simulink model "ROV_Simulation"  (R2024b)
settings: Solver=ode15s  StartTime=0.0  StopTime=120  MaxStep=0.01  MinStep=0.0001  RelTol=1e-2
PreLoadFcn:
  | load('Simulation_busses.mat')
  | 
  | %Load Initicial Conditions
  | load("InitCond.mat");
  | nu_Init = [nu_IC.u nu_IC.v nu_IC.w nu_IC.p nu_IC.q nu_IC.r];
  | eta_Init = [eta_IC.N eta_IC.E eta_IC.D eta_IC.phi eta_IC.theta eta_IC.psi];
  | 
  | %Load simulator preferences
  | load("simparam.mat");
  | %Load Thruster settings
  | load("TAM.mat");
  | load("thrusterLimit.mat");
  | %Load External forces
  | load("External_forces.mat");
  | %Load sensors
  | load("Dependencies\Workspaces\Sensors.mat");
PostLoadFcn:
  | %Define Mask for Equation of Motion
  | mask = Simulink.Mask.get('ROV_Simulation/Equation of Motion');
  | 
  | %Set options for Robots
  | robots = mask.getParameter('Robots');
  | robots.TypeOptions = who("-file","Robots.mat");
  | 
  | %Set Options for Environments
  | environments = mask.getParameter('Environments');
  | environments.TypeOptions = who("-file","Environments.mat");
  | 
  | %Set path to VR World in VR Tracer
  | %set_param('ROV_Simulation/eta2VR/VR Tracer','VrmlFile',which('Good_world_BlueRov.x3d'));
  | %set_param('ROV_Simulation/Reference Generator/VR Tracer','VrmlFile',which('Good_world_BlueRov.x3d'));
  | %set_param('ROV_Simulation/VR Sink','WorldFileName',which('Good_world_BlueRov.x3d'))
InitFcn:
  | % load local location of 3d world
  | vrFile = fullfile(pwd, 'Dependencies', 'Animation', 'Good_world_BlueRov.x3d'); % Construct the path
  | set_param('ROV_Simulation/VR Sink', 'WorldFileName', vrFile); % Update the block's parameter
  | 
  | load('Simulation_busses.mat');
  | %Load simulator preferences
  | load("simparam.mat");
  | 
  | %Load Thruster settings
  | load("TAM.mat");
  | load("thrusterLimit.mat");
  | 
  | %Load External forces
  | load("External_forces.mat");
  | 
  | %Load Reference Case study
  | load("Reference_trajectory_case_study.mat");
  | 
  | % COMMENTED OUT BY ETU 13/8-24
  | %if(not(isMATLABReleaseOlderThan("R2021b")))
  | %set_param('ROV_Simulation/External Forces/Tether','commented',double(not(Sim_param.extTetUse)))
  | %set_param('ROV_Simulation/External Forces/Manual disturbance','commented',double(not(Sim_param.extManUse)))
  | %end
  | 
  | %Load sensors
  | load("Dependencies\Workspaces\Sensors.mat");
  | noise = NoiseSelection();
  | 
  | %Load Initicial Conditions
  | load("InitCond.mat");
  | nu_Init = [nu_IC.u nu_IC.v nu_IC.w nu_IC.p nu_IC.q nu_IC.r];
  | eta_Init = [eta_IC.N eta_IC.E eta_IC.D eta_IC.phi eta_IC.theta eta_IC.psi];
  | 
  | % Load Physical parameters:
  | if(get_param('ROV_Simulation/Equation of Motion','use_app_parameters') == "on")
  |     %env = get_param('ROV_Simulation/Equation of Motion','Environments');
  |     %env = str2sym(env);
  |     %env = sprintf("%s",env);
  |     Env = LoadEnvironment(Sim_param.Env);
  | end
  | 
  | % Load robot chosen in "Equation of Motion" block:
  | if(get_param('ROV_Simulation/Equation of Motion','use_app_parameters') == "on")
  |     %rob = get_param('ROV_Simulation/Equation of Motion','Robots');
  |     %rob = str2sym(rob);
  |     %rob = sprintf("%s",rob);
  |     Robot = LoadRobotParameters(Sim_param.Robot);
  | else
  |     ROV_Constants;
  |     Robot = ROV_Struct2Bus(ROV);
  | end
  | 
  | % Pos Rov
  | xi = eta_Init(1);
  | yi = eta_Init(2);
  | zi = eta_Init(3);
  | 
  | knots = 11;
  | len_tet = 35;
  | ln_tet = len_tet/(knots-1);
  | z_tet_i = 0;
  | z1 = zi/2-ln_tet;
  | 
  | len_1 = len_tet/2-ln_tet;
  | x_tet=zeros(knots,1);
  | z_tet=zeros(knots,1);
  | if zi == len_tet
  |     z_tet = linspace(0,zi,knots)';
  | else
  | x1 = sqrt(len_1^2-z1^2);
  | a1 = (z1-z_tet_i)/(x1-0);
  | x_inc = x1/((knots-1)/2-1);
  | 
  | for i=2:1:(knots-1)/2
  | x_tet(i,1) = x_inc*(i-1);
  | end
  | z_tet(1:(knots-1)/2,1) = a1*x_tet(1:(knots-1)/2,1);
  | z2 = zi/2+ln_tet ;
  | x2 = sqrt(len_1^2-z1^2);
  | a2 = (zi-z2)/(0-x2);
  | x_tet((knots+1)/2:(knots-1)/2+2,1) = x2;
  | z_tet((knots+1)/2) = z2-ln_tet;
  | for i=(knots-1)/2+2:1:knots-1
  | x_tet(i+1,1) = x2-x_inc*(i-((knots+1)/2));
  | end
  | x_tet((knots-1)/2+1:(knots-1)/2+2,1) = x2;
  | z_tet((knots+1)/2+1:end,1) = a2*x_tet((knots+1)/2+1:end,1)+zi;
  | end
  | x_tet = x_tet+xi;
  | P10=[xi yi zi]';
  | P9=[x_tet(10) yi z_tet(10) ]';
  | P8=[x_tet(9) yi z_tet(9) ]';
  | P7=[x_tet(8)  yi z_tet(8) ]';
  | P6=[x_tet(7) yi z_tet(7)]';
  | P5=[(x_tet(6)) yi z_tet(6)]';
  | P4=[x_tet(5) yi z_tet(5)]';
  | P3=[x_tet(4) yi z_tet(4)]';
  | P2=[x_tet(3) yi z_tet(3)]';
  | P1=[x_tet(2) yi z_tet(2)]';
  | P0=[xi yi 0]';
  | 
  | 
  | x0 = 0;
  | y0 = -5;
  | z0 = 5;
  | Vr = 0.5;
  | R = 10;
  | total_time = 120;
  | dt = 0.0001;
  | 
  | [path, t_path] = pathgen(Vr, R, x0, y0, z0, total_time, dt);
  | 
  | % Enregistrer séparément
  | save('C:\Users\smaha\OneDrive\Bureau\ROVSimulator2024a\Parameters\path.mat', 'path');
  | save('C:\Users\smaha\OneDrive\Bureau\ROVSimulator2024a\Parameters\t_path.mat', 't_path');
  | 
  | % Charger dans l’espace de travail de base
  | %tmp1 = load('C:\Users\smaha\OneDrive\Bureau\ROVSimulator2024a\Parameters\path.mat');
  | %tmp2 = load('C:\Users\smaha\OneDrive\Bureau\ROVSimulator2024a\Parameters\t_path.mat');
  | 
  | %assignin('base', 'path', tmp1.path);
  | %assignin('base', 't_path', tmp2.t_path);
undefined symbols (not defined in the file or in its folder): eta_IC, EXT, nu_IC, Sim_param, T, T_inv, thrustLowerLimit, thrustUpperLimit
libraries: vrlib x2
(part 1 of 2)
settings Model: Creator=GT82BC  LastModifiedBy=smaha
settings SolverCC: InitialStep=0.0001  MaxOrder=2  MaxConsecutiveZCs=10^6  MaxConsecutiveMinStep=100  AlgebraicLoopSolver=TrustRegion
settings DebuggingCC: InheritedTsInSrcMsg=none  UnconnectedInputMsg=warning  UnconnectedOutputMsg=warning  UnconnectedLineMsg=warning  BusObjectLabelMismatch=none
settings ModelReferenceCC: EnableRefExpFcnMdlSchedulingChecks=on
settings RTWCC: GPUComputeCapability=3.5  DLArmComputeVersion=19.05
settings ConfigComp: CovScope=ReferencedModels

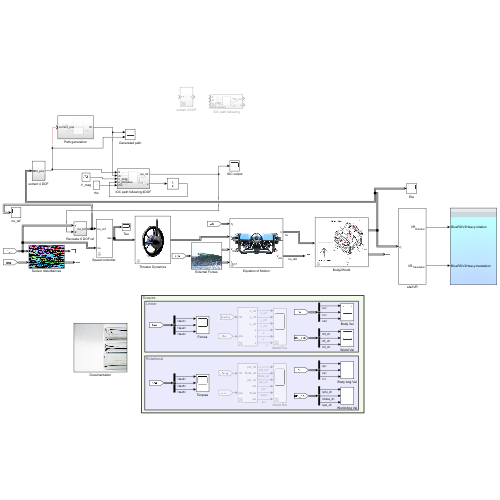
[thumbnail: Simulink's stored preview of the top-level diagram]
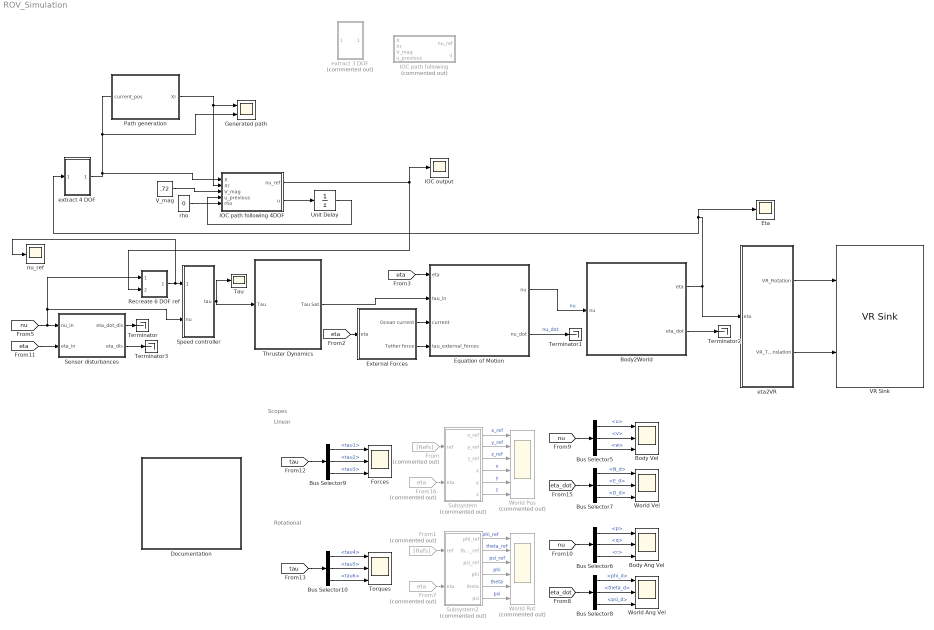
== system ROV_Simulation ==
  note: Rotational
  note: Linear
  note: Scopes
  Body Ang Vel : Scope  NumInputPorts=3
  Body Vel : Scope  NumInputPorts=3
  Body2World : Subsystem  mask(Body-Fixed to Earth-Fixed){InitialCondition=eta_Init}
      mask prompts: InitialCondition "Simulink:blkprm_prompts:IntegratorInitCond"
      mask Help: winopen('Documentation\ROVmodel.pdf')
      mask DialogControl InitialCondition Prompt: Initial condition:
      mask Display: image('BlueROV1.png')
  Bus Selector10 : BusSelector  OutputSignals=tau4,tau5,tau6
  Bus Selector5 : BusSelector  OutputSignals=u,v,w
  Bus Selector6 : BusSelector  OutputSignals=p,q,r
  Bus Selector7 : BusSelector  OutputSignals=N_d,E_d,D_d
  Bus Selector8 : BusSelector  OutputSignals=phi_d,theta_d,psi_d
  Bus Selector9 : BusSelector  OutputSignals=tau1,tau2,tau3
  Documentation : Subsystem
      settings: OpenFcn="open(\"Documentation\\ROVmodel.pdf\");"
      mask Display: image('documentation.jpg'); % Use "Edit Image" on toolstrip to change.
  Equation of Motion : Subsystem  mask(Equation of Motion){InitialCondition=nu_Init, use_app_parameters=on, Robots=BlueROV2_Heavy, Environments=Offshore}
      mask prompts: InitialCondition "Simulink:blkprm_prompts:IntegratorInitCond"; use_app_parameters "Use parameters from App"; Robots "Robot"; Environments "Environment"
      mask init:
      | mask = Simulink.Mask.get('ROV_Simulation/Equation of Motion');
      | btn = mask.getDialogControl('mscript_btn');
      | app_panel = mask.getDialogControl('app_parameter_panel');
      | mscript_panel = mask.getDialogControl('mscript_parameter_panel');
      | if(get_param('ROV_Simulation/Equation of Motion','use_app_parameters') == "off")
      |     app_panel.Visible = 'off';
      |     mscript_panel.Visible = 'on';
      | else
      |     app_panel.Visible = 'on';
      |     mscript_panel.Visible = 'off';
      | end
      mask TypeOptions InitialCondition: Integrator/InitialCondition
      mask Callback use_app_parameters:
      | mask = Simulink.Mask.get('ROV_Simulation/Equation of Motion');
      | btn = mask.getDialogControl('mscript_btn');
      | app_panel = mask.getDialogControl('app_parameter_panel');
      | mscript_panel = mask.getDialogControl('mscript_parameter_panel');
      | if(get_param('ROV_Simulation/Equation of Motion','use_app_parameters') == "off")
      |     app_panel.Visible = 'off';
      |     mscript_panel.Visible = 'on';
      | else
      |     app_panel.Visible = 'on';
      |     mscript_panel.Visible = 'off';
      | end
      mask TypeOptions Robots: BlueROV2_Heavy
      mask TypeOptions Environments: Lab | Offshore
      mask DialogControl InitialCondition Prompt: Initial condition:
      mask DialogControl refresh_options Prompt: Refresh Options
      mask DialogControl refresh_options Callback:
      | %Define Mask for Equation of Motion
      | mask = Simulink.Mask.get('ROV_Simulation/Equation of Motion');
      | 
      | %Set options for Robots
      | robots = mask.getParameter('Robots');
      | robots.TypeOptions = who("-file","Robots.mat");
      | 
      | %Set Options for Environments
      | environments = mask.getParameter('Environments');
      | environments.TypeOptions = who("-file","Environments.mat");
      mask DialogControl Robot_script_btn Prompt: ROV Parameters
      mask DialogControl Robot_script_btn Callback: open('ROV_Constants.m');
      mask DialogControl Environment_script_btn Prompt: Environmental Parameters
      mask DialogControl Environment_script_btn Callback: open('Environmental_Constants.m');
      mask DialogControl ChangeGroup Prompt: Change Parameters
      mask DialogControl Change_Parameters Prompt: Open App
      mask DialogControl Change_Parameters Callback: ROV_App;
      mask Display:
      | image('$imagefile'); % Use "Edit Image" on toolstrip to change.
      | 
      | port_label('input',1,'\eta','texmode', 'on')
      | port_label('input',2,'\tau_{in}','texmode', 'on')
      | port_label('input',3,'\nu_{Water}','texmode', 'on')
      | port_label('input',4,'\tau_{EXT}','texmode', 'on')
      | 
      | 
      | port_label('output',1,'\nu','texmode', 'on')
      | port_label('output',2,'\nu_{dot}','texmode','on')
  Eta : Scope
  External Forces : Subsystem
      mask Display: image('Waves.jpg')
  Forces : Scope  NumInputPorts=3
  From : From  Commented=on  GotoTag=Refs
  From1 : From  Commented=on  GotoTag=Refs
  From16 : From  Commented=on  GotoTag=eta  TagVisibility=global
  From7 : From  Commented=on  GotoTag=eta  TagVisibility=global
  Generated path : Scope  NumInputPorts=2
  IOC output : Scope
  IOC path following : Subsystem  Commented=on
  IOC path following 4DOF : Subsystem
  Path generation : Subsystem
  Recreate 6 DOF ref : Subsystem
  Sensor disturbances : Subsystem
      mask Display: image('Noise.jpg')
  "Speed controller " : Subsystem  mask{Kp=10, Ki=10, Kd=0}
  Subsystem : Subsystem  Commented=on
  Subsystem2 : Subsystem  Commented=on
  Tau : Scope
  Terminator{,1..3} (4 alike) : Terminator
  Thruster Dynamics : Subsystem  mask(Thruster Dynamics){}
      mask help: Thruster Dynamics & Saturation
      mask Display: image('Thruster11.jpg')
  Torques : Scope  NumInputPorts=3
  Unit Delay : UnitDelay  SampleTime=-1  HasFrameUpgradeWarning=on
  VR Sink : vrlib/VR Sink  InstantiateOnLoad=on  SampleTime=0.1  ViewEnable=on  RemoteChange=off  RemoteView=off  FieldsWritten=BlueROV2Heavy.rotation.4.1.1.double#BlueROV2Heavy.translation.3.1.1.double  WorldFileName=C:\Users\smaha\OneDrive\Bureau\ROVSimulator2024a\Dependencies\Animation\Good_world_BlueRov.x3d  AutoView=on  VideoDimensions=[]  AllowVariableSize=off  FigureProperties="{'Position', 'Name', 'Viewpoint', 'CameraBound', 'CameraDirection', 'CameraPosition', 'CameraUpVector', 'ZoomFactor', 'Antialiasing', 'Headlight', 'Lighting', 'Sound', 'StatusBar', 'Stereo3D', 'Stereo3DCameraOffset', 'Stereo3DHIT', 'ToolBar', 'Textures', 'Transparency', 'Triad', 'Wireframe', 'NavZones', 'NavMode', 'NavSpeed', 'CaptureFileFormat', 'CaptureFileName', 'Record2DCompressMethod', 'Record2DCompressQuality', 'Record2D', 'Record2DFileName', 'Record2DFPS', 'NavPanel'; [1.0000000000000000e+00   7.4199999999999932e+01   1.5360000000000000e+03   6.9080000000000007e+02], '', 'FixedtoROV', 'on', [3.1410759951924716e-02   0.0000000000000000e+00  -9.9950656033827134e-01], [3.7692911942309659e-01   0.0000000000000000e+00   2.0059212759407377e+00], [0.0000000000000000e+00   1.0000000000000000e+00   0.0000000000000000e+00], [1.0000000000000000e+00], 'on', 'on', 'on', 'on', 'on', 'off', [1.0000000149011612e-01], [0.0000000000000000e+00], 'on', 'on', 'on', 'bottomright', 'off', 'off', 'walk', 'normal', 'tif', '%f_anim_%n.tif', 'auto', [7.5000000000000000e+01], 'off', '%f_anim_%n.avi', 'auto', 'halfbar'}"
  V_mag : Constant  Value=.72
  World Ang Vel : Scope  NumInputPorts=3
  World Pos : Scope  Commented=on  NumInputPorts=6
  World Rot : Scope  Commented=on  NumInputPorts=6
  World Vel : Scope  NumInputPorts=3
  eta2VR : Subsystem
      mask Display:
      | port_label('input',1,'\eta','texmode', 'on')
      | 
      | port_label('output',1,'VR_{Rotation}','texmode', 'on')
      | port_label('output',2,'VR_{Translation}','texmode','on')
  extract 3 DOF : Subsystem  Commented=on
  extract 4 DOF : Subsystem
  nu_ref : Scope
  rho : Constant  Value=0
  wires:
  Equation of Motion/2(nu_dot) -> Terminator1/1  "nu_dot"
  Bus Selector8/1 -> World Ang Vel/1  "<phi_d>"
  Bus Selector10/2 -> Torques/2  "<tau5>"
  Bus Selector8/2 -> World Ang Vel/2  "<theta_d>"
  [tau] -> Bus Selector9/1
  Bus Selector8/3 -> World Ang Vel/3  "<psi_d>"
  [nu] -> Bus Selector6/1
  Bus Selector5/3 -> Body Vel/3  "<w>"
  Bus Selector10/3 -> Torques/3  "<tau6>"
  [eta_dot] -> Bus Selector8/1
  Bus Selector7/1 -> World Vel/1  "<N_d>"
  [eta_dot] -> Bus Selector7/1
  Bus Selector6/3 -> Body Ang Vel/3  "<r>"
  Bus Selector6/1 -> Body Ang Vel/1  "<p>"
  Bus Selector5/1 -> Body Vel/1  "<u>"
  Bus Selector7/3 -> World Vel/3  "<D_d>"
  Bus Selector5/2 -> Body Vel/2  "<v>"
  Bus Selector9/2 -> Forces/2  "<tau2>"
  Bus Selector7/2 -> World Vel/2  "<E_d>"
  Bus Selector10/1 -> Torques/1  "<tau4>"
  [nu] -> Bus Selector5/1
  Bus Selector6/2 -> Body Ang Vel/2  "<q>"
  Bus Selector9/1 -> Forces/1  "<tau1>"
  Bus Selector9/3 -> Forces/3  "<tau3>"
  From16/1 -> Subsystem/2(eta)
  [tau] -> Bus Selector10/1
  From/1 -> Subsystem/1(ref)
  Subsystem/1(x_ref) -> World Pos/1  "x_ref"
  Subsystem/2(y_ref) -> World Pos/2  "y_ref"
  Subsystem/3(z_ref) -> World Pos/3  "z_ref"
  Subsystem/4(x) -> World Pos/4  "x"
  Subsystem/5(y) -> World Pos/5  "y"
  Subsystem/6(z) -> World Pos/6  "z"
  From7/1 -> Subsystem2/2(eta)
  From1/1 -> Subsystem2/1(ref)
  Subsystem2/1(phi_ref) -> World Rot/1  "phi_ref"
  Subsystem2/2(theta_ref) -> World Rot/2  "theta_ref"
  Subsystem2/3(psi_ref) -> World Rot/3  "psi_ref"
  Subsystem2/4(phi) -> World Rot/4  "phi"
  Subsystem2/5(theta) -> World Rot/5  "theta"
  Subsystem2/6(psi) -> World Rot/6  "psi"
  [eta] -> Equation of Motion/1(eta)
  [eta] -> Sensor disturbances/2(eta_in)
  eta2VR/1(VR_Rotation) -> VR Sink/1
  eta2VR/2(VR_Translation) -> VR Sink/2
  Thruster Dynamics/1(Tau Sat) -> Equation of Motion/2(tau_in)
  [eta] -> External Forces/1(eta)
  External Forces/1(Ocean current) -> Equation of Motion/3(current)
  External Forces/2(Tether force) -> Equation of Motion/4(tau_external_forces)
  Equation of Motion/1(nu) -> Body2World/1(nu)  "nu"
  Body2World/2(eta_dot) -> Terminator2/1
  Body2World/1(eta) -> eta2VR/1(eta), Eta/1, extract 4 DOF/1(In1)
  V_mag/1 -> IOC path following 4DOF/3(V_mag)
  Path generation/1(Xr) -> IOC path following 4DOF/2(Xr), Generated path/1
  extract 4 DOF/1(current_pos) -> Generated path/2, IOC path following 4DOF/1(X)
  IOC path following 4DOF/1(nu_ref) -> IOC output/1, Recreate 6 DOF ref/2(In2)
  Recreate 6 DOF ref/1(nu_ref) -> nu_ref/1, "Speed controller "/1(nu_ref)
  IOC path following 4DOF/2(u) -> Unit Delay/1
  Unit Delay/1 -> IOC path following 4DOF/4(u_previous)
  Sensor disturbances/1(eta_dot_dis) -> Terminator/1
  "Speed controller "/1(tau) -> Thruster Dynamics/1(Tau), Tau/1
  [nu] -> Recreate 6 DOF ref/1(In1), "Speed controller "/2(nu), Sensor disturbances/1(nu_in)
  Sensor disturbances/2(eta_dis) -> Terminator3/1
  rho/1 -> IOC path following 4DOF/5(rho)

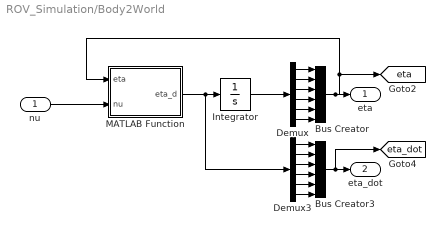
== system ROV_Simulation/Body2World ==
  nu : Inport  OutDataTypeStr=Bus: nu
  Bus Creator : BusCreator  Inputs=6  OutDataTypeStr=Bus: eta
      settings: InheritFromInputs=off
  Bus Creator3 : BusCreator  Inputs=6  OutDataTypeStr=Bus: eta_d
      settings: InheritFromInputs=off
  Demux : Demux  Outputs=6
  Demux3 : Demux  Outputs=6
  Integrator : Integrator  InitialCondition=InitialCondition
  MATLAB Function : MATLAB Function  TreatAsAtomicUnit=on
  eta : Outport  OutDataTypeStr=Bus: eta
  eta_dot : Outport  Port=2  OutDataTypeStr=Bus: eta_d
  tag scope: eta=global, eta_dot=global
  wires:
  Demux3/3 -> Bus Creator3/3
  MATLAB Function/1(eta_d) -> Demux3/1, Integrator/1
  Demux3/4 -> Bus Creator3/4
  Demux/4 -> Bus Creator/4
  Demux/5 -> Bus Creator/5
  Demux/1 -> Bus Creator/1
  Integrator/1 -> Demux/1
  Demux/3 -> Bus Creator/3
  Demux3/1 -> Bus Creator3/1
  Demux3/6 -> Bus Creator3/6
  Demux/6 -> Bus Creator/6
  Demux3/2 -> Bus Creator3/2
  Demux/2 -> Bus Creator/2
  Demux3/5 -> Bus Creator3/5
  Bus Creator/1 -> [eta], MATLAB Function/1(eta), eta/1
  Bus Creator3/1 -> [eta_dot], eta_dot/1
  nu/1 -> MATLAB Function/2(nu)

== system ROV_Simulation/Documentation ==

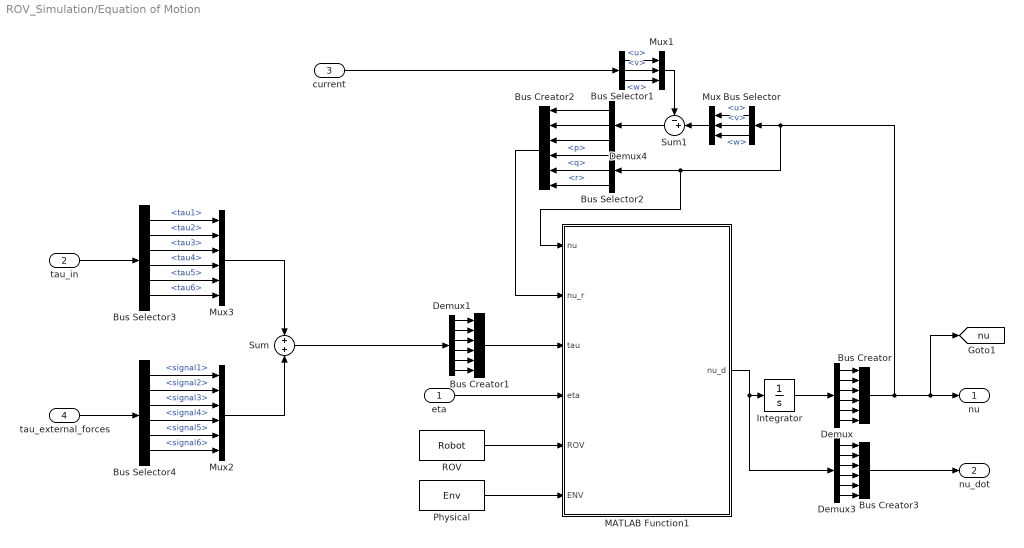
== system ROV_Simulation/Equation of Motion ==
  eta : Inport  OutDataTypeStr=Bus: eta
  tau_in : Inport  Port=2  OutDataTypeStr=Bus: tau
  current : Inport  Port=3  OutDataTypeStr=Bus: V_water
  tau_external_forces : Inport  Port=4  OutDataTypeStr=Bus: tau
  Bus Creator : BusCreator  Inputs=6  OutDataTypeStr=Bus: nu
      settings: InheritFromInputs=off
  Bus Creator1 : BusCreator  Inputs=6  OutDataTypeStr=Bus: tau
      settings: InheritFromInputs=off
  Bus Creator2 : BusCreator  Inputs=6  OutDataTypeStr=Bus: nu
      settings: InheritFromInputs=off
  Bus Creator3 : BusCreator  Inputs=6  OutDataTypeStr=Bus: nu_d
      settings: InheritFromInputs=off
  Bus Selector : BusSelector  OutputSignals=u,v,w
  Bus Selector1 : BusSelector  OutputSignals=u,v,w
  Bus Selector2 : BusSelector  OutputSignals=p,q,r
  Bus Selector3 : BusSelector  OutputSignals=tau1,tau2,tau3,tau4,tau5,tau6
  Bus Selector4 : BusSelector  OutputSignals=signal1,signal2,signal3,signal4,signal5,signal6
  Demux : Demux  Outputs=6
  Demux1 : Demux  Outputs=6
  Demux3 : Demux  Outputs=6
  Demux4 : Demux  Outputs=3
  Integrator : Integrator  InitialCondition=InitialCondition
  MATLAB Function1 : MATLAB Function  TreatAsAtomicUnit=on
  Mux : Mux  Inputs=3
  Mux1 : Mux  Inputs=3
  Mux2 : Mux  Inputs=6
  Mux3 : Mux  Inputs=6
  Physical : Constant  Value=Env  OutDataTypeStr=Bus: ENV_bus
  ROV : Constant  Value=Robot  OutDataTypeStr=Bus: ROV_bus
  Sum : Sum
  Sum1 : Sum  Inputs=-+|
  nu : Outport  OutDataTypeStr=Bus: nu
  nu_dot : Outport  Port=2  OutDataTypeStr=Bus: nu_d
  tag scope: nu=global
  wires:
  Demux3/3 -> Bus Creator3/3
  Demux/4 -> Bus Creator/4
  Demux3/4 -> Bus Creator3/4
  MATLAB Function1/1(nu_d) -> Demux3/1, Integrator/1
  Demux3/1 -> Bus Creator3/1
  Demux/5 -> Bus Creator/5
  Demux3/6 -> Bus Creator3/6
  Bus Creator3/1 -> nu_dot/1
  Physical/1 -> MATLAB Function1/6(ENV)
  Demux3/2 -> Bus Creator3/2
  Demux3/5 -> Bus Creator3/5
  Demux/2 -> Bus Creator/2
  Integrator/1 -> Demux/1
  Demux/1 -> Bus Creator/1
  Demux/3 -> Bus Creator/3
  Demux/6 -> Bus Creator/6
  eta/1 -> MATLAB Function1/4(eta)
  ROV/1 -> MATLAB Function1/5(ROV)
  Bus Creator1/1 -> MATLAB Function1/3(tau)
  Demux1/5 -> Bus Creator1/5
  Demux1/6 -> Bus Creator1/6
  Demux1/1 -> Bus Creator1/1
  Demux1/2 -> Bus Creator1/2
  Demux1/3 -> Bus Creator1/3
  Demux1/4 -> Bus Creator1/4
  Bus Creator/1 -> MATLAB Function1/1(nu), Bus Selector2/1, Bus Selector/1, [nu], nu/1
  Mux/1 -> Sum1/2
  Sum1/1 -> Demux4/1
  Demux4/1 -> Bus Creator2/1
  Demux4/2 -> Bus Creator2/2
  Demux4/3 -> Bus Creator2/3
  Bus Selector1/1 -> Mux1/1  "<u>"
  Bus Selector1/2 -> Mux1/2  "<v>"
  Bus Selector1/3 -> Mux1/3  "<w>"
  Mux1/1 -> Sum1/1
  Bus Selector/1 -> Mux/1  "<u>"
  Bus Selector/2 -> Mux/2  "<v>"
  Bus Selector/3 -> Mux/3  "<w>"
  current/1 -> Bus Selector1/1
  Bus Selector2/3 -> Bus Creator2/6  "<r>"
  Bus Selector2/2 -> Bus Creator2/5  "<q>"
  Bus Selector2/1 -> Bus Creator2/4  "<p>"
  Bus Creator2/1 -> MATLAB Function1/2(nu_r)
  tau_in/1 -> Bus Selector3/1
  tau_external_forces/1 -> Bus Selector4/1
  Bus Selector4/1 -> Mux2/1  "<signal1>"
  Bus Selector4/2 -> Mux2/2  "<signal2>"
  Bus Selector4/3 -> Mux2/3  "<signal3>"
  Bus Selector4/4 -> Mux2/4  "<signal4>"
  Bus Selector4/5 -> Mux2/5  "<signal5>"
  Bus Selector4/6 -> Mux2/6  "<signal6>"
  Bus Selector3/1 -> Mux3/1  "<tau1>"
  Bus Selector3/2 -> Mux3/2  "<tau2>"
  Bus Selector3/3 -> Mux3/3  "<tau3>"
  Bus Selector3/4 -> Mux3/4  "<tau4>"
  Bus Selector3/5 -> Mux3/5  "<tau5>"
  Bus Selector3/6 -> Mux3/6  "<tau6>"
  Mux3/1 -> Sum/1
  Mux2/1 -> Sum/2
  Sum/1 -> Demux1/1

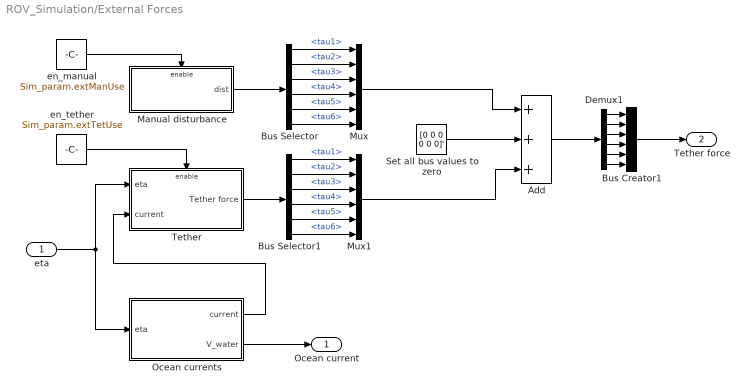
== system ROV_Simulation/External Forces ==
  eta : Inport
  Add : Sum  Inputs=+++
  Bus Creator1 : BusCreator  Inputs=6  OutDataTypeStr=Bus: tau
  Bus Selector : BusSelector  OutputSignals=tau1,tau2,tau3,tau4,tau5,tau6
  Bus Selector1 : BusSelector  OutputSignals=tau1,tau2,tau3,tau4,tau5,tau6
  Demux1 : Demux  Outputs=6
  Manual disturbance : Subsystem  TreatAsAtomicUnit=on
  Mux : Mux  Inputs=6
  Mux1 : Mux  Inputs=6
  Ocean currents : Subsystem  TreatAsAtomicUnit=on
  Set all bus values to zero : Constant  Value=[0 0 0 0 0 0]'
  Tether : Subsystem  TreatAsAtomicUnit=on
  en_manual : Constant  Value=Sim_param.extManUse
  en_tether : Constant  Value=Sim_param.extTetUse
  Ocean current : Outport
  Tether force : Outport  Port=2
  wires:
  eta/1 -> Ocean currents/1(eta), Tether/1(eta)
  Manual disturbance/1(dist) -> Bus Selector/1
  Demux1/4 -> Bus Creator1/4
  Demux1/2 -> Bus Creator1/2
  Demux1/5 -> Bus Creator1/5
  Demux1/1 -> Bus Creator1/1
  Bus Creator1/1 -> Tether force/1
  Demux1/6 -> Bus Creator1/6
  Demux1/3 -> Bus Creator1/3
  Ocean currents/1(current) -> Tether/2(current)
  Ocean currents/2(V_water) -> Ocean current/1
  en_tether/1 -> Tether/Enable
  en_manual/1 -> Manual disturbance/Enable
  Tether/1(Tether force) -> Bus Selector1/1
  Bus Selector1/1 -> Mux1/1  "<tau1>"
  Bus Selector1/2 -> Mux1/2  "<tau2>"
  Bus Selector1/3 -> Mux1/3  "<tau3>"
  Bus Selector1/4 -> Mux1/4  "<tau4>"
  Bus Selector1/5 -> Mux1/5  "<tau5>"
  Bus Selector1/6 -> Mux1/6  "<tau6>"
  Bus Selector/1 -> Mux/1  "<tau1>"
  Bus Selector/2 -> Mux/2  "<tau2>"
  Bus Selector/3 -> Mux/3  "<tau3>"
  Bus Selector/4 -> Mux/4  "<tau4>"
  Bus Selector/5 -> Mux/5  "<tau5>"
  Bus Selector/6 -> Mux/6  "<tau6>"
  Set all bus values to zero/1 -> Add/2
  Mux/1 -> Add/1
  Mux1/1 -> Add/3
  Add/1 -> Demux1/1

== system ROV_Simulation/IOC path following ==
  X : Inport
  Xr : Inport  Port=2
  V_mag : Inport  Port=3
  u_previous : Inport  Port=4
  IOC path following : MATLAB Function  TreatAsAtomicUnit=on
  Scope : Scope  NumInputPorts=2
  nu_ref : Outport
  u : Outport  Port=2
  wires:
  IOC path following/1(nu_ref) -> nu_ref/1
  X/1 -> IOC path following/1(X)
  Xr/1 -> IOC path following/2(Xr)
  V_mag/1 -> IOC path following/3(V_mag)
  u_previous/1 -> IOC path following/4(u_previous)
  IOC path following/2(u) -> u/1
  IOC path following/3(e2) -> Scope/1
  IOC path following/4(e3) -> Scope/2

== system ROV_Simulation/IOC path following 4DOF ==
  X : Inport
  Xr : Inport  Port=2
  V_mag : Inport  Port=3
  u_previous : Inport  Port=4
  rho : Inport  Port=5
  Errors : Scope  NumInputPorts=3
  IOC path following 4 DOF : MATLAB Function  TreatAsAtomicUnit=on
  nu_ref : Outport
  u : Outport  Port=2
  wires:
  IOC path following 4 DOF/1(nu_ref) -> nu_ref/1
  X/1 -> IOC path following 4 DOF/1(X)
  Xr/1 -> IOC path following 4 DOF/2(Xr)
  V_mag/1 -> IOC path following 4 DOF/3(V_mag)
  IOC path following 4 DOF/3(e2) -> Errors/1
  IOC path following 4 DOF/4(e3) -> Errors/2
  IOC path following 4 DOF/2(u) -> u/1
  u_previous/1 -> IOC path following 4 DOF/4(u_previous)
  IOC path following 4 DOF/5(e4) -> Errors/3
  rho/1 -> IOC path following 4 DOF/5(rho)

== system ROV_Simulation/Path generation ==
  current_pos : Inport
  Clock : Clock
  Reference selector nearest point : MATLAB Function  Commented=on  TreatAsAtomicUnit=on
  Reference selector time sensitive : MATLAB Function  TreatAsAtomicUnit=on
  Terminator : Terminator
  Xr : Outport
  wires:
  Reference selector time sensitive/1(Xr) -> Xr/1
  Clock/1 -> Reference selector time sensitive/1(current_time)
  current_pos/1 -> Terminator/1

== system ROV_Simulation/Recreate 6 DOF ref ==
  In1 : Inport
  In2 : Inport  Port=2
  Bus Creator : BusCreator  Inputs=6  OutDataTypeStr=Bus: nu  NonVirtualBus=on
      settings: InheritFromInputs=off
  Bus Selector : BusSelector  OutputSignals=p,q
  Demux : Demux
  nu_ref : Outport
  wires:
  Demux/1 -> Bus Creator/1  "u"
  In2/1 -> Demux/1
  In1/1 -> Bus Selector/1
  Bus Creator/1 -> nu_ref/1
  Demux/2 -> Bus Creator/2  "v"
  Bus Selector/2 -> Bus Creator/5  "<q>"
  Bus Selector/1 -> Bus Creator/4  "<p>"
  Demux/4 -> Bus Creator/6
  Demux/3 -> Bus Creator/3  "r"
